simulink library "TripedLibraryMAX"  (R2021a)
settings: Solver=VariableStepAuto  StartTime=0.0  StopTime=10.0  RelTol=1e-3
undefined symbols (not defined in the file or in its folder): X, Y, Z
libraries: sm_lib x100, nesl_utility x31, $bdroot x27, robotlib x27, simulink x6
(part 6 of 6)

== matlab function TripedLibraryMAX/Simplified Model/Simplified TriPed (Kinematics)/Forward Kinematics/MATLAB Function ==
  data: Jointstate input; leg_pos output; LegNumber input
function leg_pos = fcn(Jointstate, LegNumber)
waym = Weg0(eul2quat(Jointstate(1:3).','XYZ'));
wayLLm = AddLegLinear(waym,Jointstate(4));
legstate = getPosfromHomogenMatrix(wayLLm);
leg_pos = transformCoordinateSystem(legstate(1),legstate(2),legstate(3),LegNumber);
end




function [new_pos] = transformCoordinateSystem(oldx,oldy,oldz,legNr)
% this function translates the x,y,z coordinates from the CCS into
% coordinates from a cooridnate system, which is like the ccs but rotated
% ,such that its x axis points towards the leg. This makes the inverse
% kinematics for the leg 1 & 2 easier.

angle = -legNr * 120 * pi/180;

newCoord = [cos(angle), -sin(angle), 0, 0; sin(angle), cos(angle), 0, 0; 0, 0, 1, 0; 0, 0, 0, 1]* [oldx;oldy;oldz;1];
newx = newCoord(1);
newy = newCoord(2);
newz = newCoord(3);

new_pos=[newx;newy;newz];
end

function [Weg0Mat] = Weg0(quat)
a0=quat(1);
b0=quat(2);
c0=quat(3);
d0=quat(4);
A1 = homogenTranslationMat(0.265,0,0.014);
A2 = makeRotHomogen(quatRotM(a0,b0,c0,d0));
Weg0Mat = A1 * A2;
end



function [ALeg] = AddLegLinear(A,theta0)
% this functions adds transformations from the Point P; which is the
% Endpoint from Weg0,1,2; to the FKS at the LegLinear part.
%{
Inputs:
A: Transformationmatrix, which describes Transformation from HKS to P, Use
Weg0.m for that.
theta0: angle [radians] for joint at MKS00
%}

A1 = homogenTranslationMat(1.640, 0, -0.037); % transform P to P0 (center of LegLinear rotation)

theta0 = -1* theta0 * 0.07/(2*pi*1.5); % gear constraint: transfrom theta at MKS00 to theta around P0, gear ratio is 0.07/(2*pi*1.5) [circumferences of the gears MCS00 / LL]
startingvalue = -0 * pi/180;
theta0 = theta0 + startingvalue; %offset, depending on which position the Leg is for theta at MKS00 is 0
A2 = makeRotHomogen(quatRotM(cos(theta0/2),0,sin(theta0/2),0)); % rotate theta [radians] around y-axis of P0

A3 = homogenTranslationMat(-1.500,0,0); %transform from P0-joint to FKS

ALeg = A*A1*A2*A3;
end

function [res] = homogenTranslationMat(x,y,z)
    %this function forms a Translation about a vector x,y,z in a homogenous
    %transformation matrix res. 
    res = [1 0 0 x; 0 1 0 y; 0 0 1 z; 0 0 0 1];
end

function [res] = makeRotHomogen(R)
% this function fills a Rotationmatrix to make it a homogen transformation
% matrix
res = [R(1,1) R(1,2) R(1,3) 0; R(2,1) R(2,2) R(2,3) 0; R(3,1) R(3,2) R(3,3) 0; 0 0 0 1];
end

function [res] = quatRotM(a,b,c,d) %%Todo: use a,b,c,d as inputs
    % this function calculates the quaternion rotation matrix for given
    % vector (kx,ky,kz) and angle theta
    res = [1-2*(c^2+d^2) 2*(b*c-d*a) 2*(b*d + c*a); 2*(b*c + d*a) 1-2*(b^2+d^2) 2*(c*d - b*a); 2*(b*d-c*a) 2*(c*d+b*a) 1-2*(b^2+c^2) ];
end




function [pos] = getPosfromHomogenMatrix(A)
    % this function returns the Vector by which a homogenous
    % Transformationmatrix shifts a Point
    x = A(1,4);
    y = A(2,4);
    z = A(3,4);

    pos=[x,y,z];
end

== matlab function TripedLibraryMAX/Examples/Spider Walking/Spider Gait ==
  settings: updateMethod=DISCRETE  sampleTime=0.01
  data: foot_0 output; swing_rad input; cmd_vel input; current_foot_pos input; current_state input; foot_1 output; foot_2 output; current_foot output; next_state output
function [foot_0,foot_1,foot_2,current_foot, next_state] = fcn( swing_rad,cmd_vel,current_foot_pos, current_state)


    initial_pos_0 = [0.4    ; 0       ;  -0]*1.2 + [0   ; 0       ; -0.6];
    initial_pos_1 = [-0.2025; -0.35074;  -0]*1.2 + [0   ; 0       ; -0.6];
    initial_pos_2 = [-0.2025;  0.35074;  -0]*1.2 + [0   ; 0       ; -0.6];
    
    init_angle_0  = mod(2*pi/360* 0   + 0*pi/2,2*pi);
    init_angle_1  = mod(2*pi/360* 240 + 0*pi/2,2*pi); %angle convention other direction for some reason
    init_angle_2  = mod(2*pi/360* 120 + 0*pi/2,2*pi);
    
    
    rel_step_angle= 60;
    final_angle_0 = mod(init_angle_0+2*pi/360*rel_step_angle,2*pi);
    final_angle_1 = mod(init_angle_1+2*pi/360*rel_step_angle,2*pi);
    final_angle_2 = mod(init_angle_2+2*pi/360*rel_step_angle,2*pi);
    
    current_foot= [0;0;0;0;0;0;0;0;0;0;0;0];
    next_state=current_state;

    
    persistent angle
    if isempty(angle)
        angle = [-2*pi;-2*pi;-2*pi];
    end

    
    persistent last_step_angle
    if isempty(last_step_angle)
        last_step_angle = [0;0;0];
    end
 
    persistent last_step_vel
    if isempty(last_step_vel)
        last_step_vel = [0;0;0;0;0;0];
    end

    persistent step_pos
    if isempty(step_pos)
        step_pos = [initial_pos_0;initial_pos_1;initial_pos_2];
    end
    
    
   [foot_0,current_foot(1:4) ,next_state(1),last_step_angle(1),last_step_vel(1:2),step_pos(1:3),angle(1)]  = single_leg_state_machine(initial_pos_0,last_step_vel(1:2),last_step_angle(1),current_state(1),current_foot_pos(1:4) ,step_pos(1:3),angle(1),swing_rad,cmd_vel,final_angle_0,init_angle_0);
   [foot_1,current_foot(5:8) ,next_state(2),last_step_angle(2),last_step_vel(3:4),step_pos(4:6),angle(2)]  = single_leg_state_machine(initial_pos_1,last_step_vel(3:4),last_step_angle(2),current_state(2),current_foot_pos(5:8) ,step_pos(4:6),angle(2),swing_rad,cmd_vel,final_angle_1,init_angle_1);  
   [foot_2,current_foot(9:12),next_state(3),last_step_angle(3),last_step_vel(5:6),step_pos(7:9),angle(3)]  = single_leg_state_machine(initial_pos_2,last_step_vel(5:6),last_step_angle(3),current_state(3),current_foot_pos(9:12),step_pos(7:9),angle(3),swing_rad,cmd_vel,final_angle_2,init_angle_2);


end

function [foot,current_liftoff,next_state,current_step,last_vel,step_pos,angle]=single_leg_state_machine(initial_pos,last_vel,prev_step,current_state,foot_pos,step_pos,last_angle,swing_rad,cmd_vel,stop_angle,start_angle)

timestep    = 0.01;
increment   = pi;
angle       = last_angle+increment*timestep;

height= 0.08;




[rad_walking_dir,velocity]=cart2pol(cmd_vel(1),cmd_vel(2));
walking_dir=rad_walking_dir/(2*pi)*360;
velocity = velocity/(2*pi);%/increment;

rel_step_angle = 60;%step_time/increment/timestep;
    



move_memory=angle-prev_step;

switch (current_state)
    case 0
        init_angle    = foot_pos(4);
        final_angle   = init_angle+2*pi/360*rel_step_angle;
        s = angdiff(angle,init_angle)/angdiff(final_angle,init_angle);
       
        
        if (s<=1)
            next_state = 0;
            current_step = prev_step+2.5*0.01;
        else
            next_state =1;
            current_step = angle;

        end
        
        est_trvl=  2*abs(angdiff(final_angle,init_angle)); %max((2*pi-move_memory)/increment,0);
        final_pos    = initial_pos+swing_rad*[cos(final_angle);sin(final_angle);0] + rotz(last_vel(2))*[1;0;0]*est_trvl*last_vel(1)/2;
        foot         = targeted_step(s,height,foot_pos(1:3),final_pos);
        current_liftoff = foot_pos; 
        step_pos  = initial_pos + rotz(last_vel(2))*[1;0;0]*est_trvl*last_vel(1)/2;

        
    case 1

        foot = step_pos+swing_rad*[cos(angle);sin(angle);0]-rotz(walking_dir)*[1;0;0]*move_memory*velocity;
        if (mod(angle,2*pi) >=start_angle) &&(mod(angle,2*pi) <=stop_angle) &&  norm(step_pos+swing_rad*[cos(angle);sin(angle);0]-foot) >=0.05
            next_state =0;
        else
            next_state = 1;
        end

        current_liftoff = [foot;angle];
        current_step = prev_step;
        last_vel = [velocity;walking_dir];
        
    % initialisation case to allow the simulation to settle
    case 2
        if angle >= 0
            next_state=1;
        else
            next_state=2;    
        end
        foot = initial_pos+(2*pi+angle)/(2*pi)*swing_rad*[cos(0);sin(0);0];
        current_liftoff = [foot;0]; 
        current_step = prev_step;
        
    otherwise
         next_state      = current_state;
         
         foot            = foot_pos(1:3);
         current_liftoff = foot_pos; 
         current_step = prev_step;
end
end


function foot_position=targeted_step(s,height,start,stop)
    relative_pos  = stop-start;
    [rot,len]   = cart2pol(relative_pos(1),relative_pos(2));
    foot_position = rotz(rot/(2*pi)*360)*step(s,len,height)+start;
end

function foot_position=step(s,length,height)

z  = height*exp(-(s-0.5)^2/0.01);%0.01

x = length/(1+exp(-20*(s-0.5)));
y = 0; 

foot_position = [x; y; z];
end

== matlab function TripedLibraryMAX/Full Model/ROS/Publishers/Publisher_leg_1/leg_1_extend_joint_pub/mergeValuesIntoRosMsg_test ==
  data: blankMessage input; position input; msg output Bus: SL_Bus_rosTest_sensor_msgs_JointState
function msg = assignNestedArrayMessages(blankMessage,position)
%#codegen

% To set datatype of MSG output:
%  1. Create buses for this model: robotics.ros.createSimulinkBus(gcs)
%  1. Click "Edit Data" in  Toolstrip to open "Ports and Data Manager"
%  2. Select MSG and set datatype to "Bus: SL_Bus_<modelname>_<messageType>"
% See: 
% http://www.mathworks.com/help/simulink/ug/create-structures-in-matlab-function-blocks.html

% blankMessage (geometry_msgs/PoseArray) consists of
%          Header: [1x1 Header]
%           Poses: variable-length array of geometry_msgs/Pose
%   Poses_SL_Info: struct with fields CurrentLength, ReceivedLength
%
% Poses is an array of "geometry_msgs/Pose", which consists of
%        Position: [1x1 Point]
%     Orientation: [1x1 Quaternion]
%
% Position is "geometry_msgs/Point", which consists of
%               X: 0
%               Y: 0
%               Z: 0
%
% Orientation is "geometry_msgs/Quaternion", which consists of
%               X: 0
%               Y: 0
%               Z: 0
%               W: 0

% Set the Poses array to have size 2, and modify the first two Pose
% elements

%blankMessage.Poses_SL_Info.CurrentLength = uint32(2);
%blankMessage.Poses(1).Position.X = x;
%blankMessage.Poses(1).Position.Y = y;
%blankMessage.Poses(2).Orientation.Z = z;

blankMessage.Position_SL_Info.CurrentLength = uint32(1);
%blankMessage.Velocity_SL_Info.CurrentLength = uint32(2);
%blankMessage.Effort_SL_Info.CurrentLength = uint32(2);
blankMessage.Position(1) = position;
%blankMessage.Velocity(2) = velocity;
%blankMessage.Position(2) = effort;

msg = blankMessage;

== matlab function TripedLibraryMAX/Full Model/ROS/Publishers/Publisher_leg_0/leg_0_swing_left_joint_pub/mergeValuesIntoRosMsg_test ==
  data: blankMessage input; position input; msg output Bus: SL_Bus_rosTest_sensor_msgs_JointState
function msg = assignNestedArrayMessages(blankMessage,position)
%#codegen

% To set datatype of MSG output:
%  1. Create buses for this model: robotics.ros.createSimulinkBus(gcs)
%  1. Click "Edit Data" in  Toolstrip to open "Ports and Data Manager"
%  2. Select MSG and set datatype to "Bus: SL_Bus_<modelname>_<messageType>"
% See: 
% http://www.mathworks.com/help/simulink/ug/create-structures-in-matlab-function-blocks.html

% blankMessage (geometry_msgs/PoseArray) consists of
%          Header: [1x1 Header]
%           Poses: variable-length array of geometry_msgs/Pose
%   Poses_SL_Info: struct with fields CurrentLength, ReceivedLength
%
% Poses is an array of "geometry_msgs/Pose", which consists of
%        Position: [1x1 Point]
%     Orientation: [1x1 Quaternion]
%
% Position is "geometry_msgs/Point", which consists of
%               X: 0
%               Y: 0
%               Z: 0
%
% Orientation is "geometry_msgs/Quaternion", which consists of
%               X: 0
%               Y: 0
%               Z: 0
%               W: 0

% Set the Poses array to have size 2, and modify the first two Pose
% elements

%blankMessage.Poses_SL_Info.CurrentLength = uint32(2);
%blankMessage.Poses(1).Position.X = x;
%blankMessage.Poses(1).Position.Y = y;
%blankMessage.Poses(2).Orientation.Z = z;

blankMessage.Position_SL_Info.CurrentLength = uint32(1);
%blankMessage.Velocity_SL_Info.CurrentLength = uint32(2);
%blankMessage.Effort_SL_Info.CurrentLength = uint32(2);
blankMessage.Position(1) = position;
%blankMessage.Velocity(2) = velocity;
%blankMessage.Position(2) = effort;

msg = blankMessage;

== matlab function TripedLibraryMAX/Full Model/ROS/Publishers/Publisher_leg_1/leg_1_swing_left_joint_pub/mergeValuesIntoRosMsg_test ==
  data: blankMessage input; position input; msg output Bus: SL_Bus_rosTest_sensor_msgs_JointState
function msg = assignNestedArrayMessages(blankMessage,position)
%#codegen

% To set datatype of MSG output:
%  1. Create buses for this model: robotics.ros.createSimulinkBus(gcs)
%  1. Click "Edit Data" in  Toolstrip to open "Ports and Data Manager"
%  2. Select MSG and set datatype to "Bus: SL_Bus_<modelname>_<messageType>"
% See: 
% http://www.mathworks.com/help/simulink/ug/create-structures-in-matlab-function-blocks.html

% blankMessage (geometry_msgs/PoseArray) consists of
%          Header: [1x1 Header]
%           Poses: variable-length array of geometry_msgs/Pose
%   Poses_SL_Info: struct with fields CurrentLength, ReceivedLength
%
% Poses is an array of "geometry_msgs/Pose", which consists of
%        Position: [1x1 Point]
%     Orientation: [1x1 Quaternion]
%
% Position is "geometry_msgs/Point", which consists of
%               X: 0
%               Y: 0
%               Z: 0
%
% Orientation is "geometry_msgs/Quaternion", which consists of
%               X: 0
%               Y: 0
%               Z: 0
%               W: 0

% Set the Poses array to have size 2, and modify the first two Pose
% elements

%blankMessage.Poses_SL_Info.CurrentLength = uint32(2);
%blankMessage.Poses(1).Position.X = x;
%blankMessage.Poses(1).Position.Y = y;
%blankMessage.Poses(2).Orientation.Z = z;

blankMessage.Position_SL_Info.CurrentLength = uint32(1);
%blankMessage.Velocity_SL_Info.CurrentLength = uint32(2);
%blankMessage.Effort_SL_Info.CurrentLength = uint32(2);
blankMessage.Position(1) = position;
%blankMessage.Velocity(2) = velocity;
%blankMessage.Position(2) = effort;

msg = blankMessage;

== matlab function TripedLibraryMAX/Simplified Model/Simplified TriPed (Kinematics)/Forward Kinematics/MATLAB Function1 ==
  data: Jointstate input; leg_pos output; LegNumber input
function leg_pos = fcn(Jointstate, LegNumber)
waym = Weg0(eul2quat(Jointstate(1:3).','XYZ'));
wayLLm = AddLegLinear(waym,Jointstate(4));
legstate = getPosfromHomogenMatrix(wayLLm);
leg_pos = transformCoordinateSystem(legstate(1),legstate(2),legstate(3),LegNumber);
end




function [new_pos] = transformCoordinateSystem(oldx,oldy,oldz,legNr)
% this function translates the x,y,z coordinates from the CCS into
% coordinates from a cooridnate system, which is like the ccs but rotated
% ,such that its x axis points towards the leg. This makes the inverse
% kinematics for the leg 1 & 2 easier.

angle = -legNr * 120 * pi/180;

newCoord = [cos(angle), -sin(angle), 0, 0; sin(angle), cos(angle), 0, 0; 0, 0, 1, 0; 0, 0, 0, 1]* [oldx;oldy;oldz;1];
newx = newCoord(1);
newy = newCoord(2);
newz = newCoord(3);

new_pos=[newx;newy;newz];
end

function [Weg0Mat] = Weg0(quat)
a0=quat(1);
b0=quat(2);
c0=quat(3);
d0=quat(4);
A1 = homogenTranslationMat(0.265,0,0.014);
A2 = makeRotHomogen(quatRotM(a0,b0,c0,d0));
Weg0Mat = A1 * A2;
end



function [ALeg] = AddLegLinear(A,theta0)
% this functions adds transformations from the Point P; which is the
% Endpoint from Weg0,1,2; to the FKS at the LegLinear part.
%{
Inputs:
A: Transformationmatrix, which describes Transformation from HKS to P, Use
Weg0.m for that.
theta0: angle [radians] for joint at MKS00
%}

A1 = homogenTranslationMat(1.640, 0, -0.037); % transform P to P0 (center of LegLinear rotation)

theta0 = -1* theta0 * 0.07/(2*pi*1.5); % gear constraint: transfrom theta at MKS00 to theta around P0, gear ratio is 0.07/(2*pi*1.5) [circumferences of the gears MCS00 / LL]
startingvalue = -0 * pi/180;
theta0 = theta0 + startingvalue; %offset, depending on which position the Leg is for theta at MKS00 is 0
A2 = makeRotHomogen(quatRotM(cos(theta0/2),0,sin(theta0/2),0)); % rotate theta [radians] around y-axis of P0

A3 = homogenTranslationMat(-1.500,0,0); %transform from P0-joint to FKS

ALeg = A*A1*A2*A3;
end

function [res] = homogenTranslationMat(x,y,z)
    %this function forms a Translation about a vector x,y,z in a homogenous
    %transformation matrix res. 
    res = [1 0 0 x; 0 1 0 y; 0 0 1 z; 0 0 0 1];
end

function [res] = makeRotHomogen(R)
% this function fills a Rotationmatrix to make it a homogen transformation
% matrix
res = [R(1,1) R(1,2) R(1,3) 0; R(2,1) R(2,2) R(2,3) 0; R(3,1) R(3,2) R(3,3) 0; 0 0 0 1];
end

function [res] = quatRotM(a,b,c,d) %%Todo: use a,b,c,d as inputs
    % this function calculates the quaternion rotation matrix for given
    % vector (kx,ky,kz) and angle theta
    res = [1-2*(c^2+d^2) 2*(b*c-d*a) 2*(b*d + c*a); 2*(b*c + d*a) 1-2*(b^2+d^2) 2*(c*d - b*a); 2*(b*d-c*a) 2*(c*d+b*a) 1-2*(b^2+c^2) ];
end




function [pos] = getPosfromHomogenMatrix(A)
    % this function returns the Vector by which a homogenous
    % Transformationmatrix shifts a Point
    x = A(1,4);
    y = A(2,4);
    z = A(3,4);

    pos=[x,y,z];
end

== matlab function TripedLibraryMAX/Simplified Model/Simplified TriPed (Kinematics)/Forward Kinematics/MATLAB Function2 ==
  data: Jointstate input; leg_pos output; LegNumber input
function leg_pos = fcn(Jointstate, LegNumber)
waym = Weg0(eul2quat(Jointstate(1:3).','XYZ'));
wayLLm = AddLegLinear(waym,Jointstate(4));
legstate = getPosfromHomogenMatrix(wayLLm);
leg_pos = transformCoordinateSystem(legstate(1),legstate(2),legstate(3),LegNumber);
end




function [new_pos] = transformCoordinateSystem(oldx,oldy,oldz,legNr)
% this function translates the x,y,z coordinates from the CCS into
% coordinates from a cooridnate system, which is like the ccs but rotated
% ,such that its x axis points towards the leg. This makes the inverse
% kinematics for the leg 1 & 2 easier.

angle = -legNr * 120 * pi/180;

newCoord = [cos(angle), -sin(angle), 0, 0; sin(angle), cos(angle), 0, 0; 0, 0, 1, 0; 0, 0, 0, 1]* [oldx;oldy;oldz;1];
newx = newCoord(1);
newy = newCoord(2);
newz = newCoord(3);

new_pos=[newx;newy;newz];
end

function [Weg0Mat] = Weg0(quat)
a0=quat(1);
b0=quat(2);
c0=quat(3);
d0=quat(4);
A1 = homogenTranslationMat(0.265,0,0.014);
A2 = makeRotHomogen(quatRotM(a0,b0,c0,d0));
Weg0Mat = A1 * A2;
end



function [ALeg] = AddLegLinear(A,theta0)
% this functions adds transformations from the Point P; which is the
% Endpoint from Weg0,1,2; to the FKS at the LegLinear part.
%{
Inputs:
A: Transformationmatrix, which describes Transformation from HKS to P, Use
Weg0.m for that.
theta0: angle [radians] for joint at MKS00
%}

A1 = homogenTranslationMat(1.640, 0, -0.037); % transform P to P0 (center of LegLinear rotation)

theta0 = -1* theta0 * 0.07/(2*pi*1.5); % gear constraint: transfrom theta at MKS00 to theta around P0, gear ratio is 0.07/(2*pi*1.5) [circumferences of the gears MCS00 / LL]
startingvalue = -0 * pi/180;
theta0 = theta0 + startingvalue; %offset, depending on which position the Leg is for theta at MKS00 is 0
A2 = makeRotHomogen(quatRotM(cos(theta0/2),0,sin(theta0/2),0)); % rotate theta [radians] around y-axis of P0

A3 = homogenTranslationMat(-1.500,0,0); %transform from P0-joint to FKS

ALeg = A*A1*A2*A3;
end

function [res] = homogenTranslationMat(x,y,z)
    %this function forms a Translation about a vector x,y,z in a homogenous
    %transformation matrix res. 
    res = [1 0 0 x; 0 1 0 y; 0 0 1 z; 0 0 0 1];
end

function [res] = makeRotHomogen(R)
% this function fills a Rotationmatrix to make it a homogen transformation
% matrix
res = [R(1,1) R(1,2) R(1,3) 0; R(2,1) R(2,2) R(2,3) 0; R(3,1) R(3,2) R(3,3) 0; 0 0 0 1];
end

function [res] = quatRotM(a,b,c,d) %%Todo: use a,b,c,d as inputs
    % this function calculates the quaternion rotation matrix for given
    % vector (kx,ky,kz) and angle theta
    res = [1-2*(c^2+d^2) 2*(b*c-d*a) 2*(b*d + c*a); 2*(b*c + d*a) 1-2*(b^2+d^2) 2*(c*d - b*a); 2*(b*d-c*a) 2*(c*d+b*a) 1-2*(b^2+c^2) ];
end




function [pos] = getPosfromHomogenMatrix(A)
    % this function returns the Vector by which a homogenous
    % Transformationmatrix shifts a Point
    x = A(1,4);
    y = A(2,4);
    z = A(3,4);

    pos=[x,y,z];
end

== matlab function TripedLibraryMAX/Full Model/ROS/Publishers/Publisher_leg_0/leg_0_swing_right_joint_pub/mergeValuesIntoRosMsg_test ==
  data: blankMessage input; position input; msg output Bus: SL_Bus_rosTest_sensor_msgs_JointState
function msg = assignNestedArrayMessages(blankMessage,position)
%#codegen

% To set datatype of MSG output:
%  1. Create buses for this model: robotics.ros.createSimulinkBus(gcs)
%  1. Click "Edit Data" in  Toolstrip to open "Ports and Data Manager"
%  2. Select MSG and set datatype to "Bus: SL_Bus_<modelname>_<messageType>"
% See: 
% http://www.mathworks.com/help/simulink/ug/create-structures-in-matlab-function-blocks.html

% blankMessage (geometry_msgs/PoseArray) consists of
%          Header: [1x1 Header]
%           Poses: variable-length array of geometry_msgs/Pose
%   Poses_SL_Info: struct with fields CurrentLength, ReceivedLength
%
% Poses is an array of "geometry_msgs/Pose", which consists of
%        Position: [1x1 Point]
%     Orientation: [1x1 Quaternion]
%
% Position is "geometry_msgs/Point", which consists of
%               X: 0
%               Y: 0
%               Z: 0
%
% Orientation is "geometry_msgs/Quaternion", which consists of
%               X: 0
%               Y: 0
%               Z: 0
%               W: 0

% Set the Poses array to have size 2, and modify the first two Pose
% elements

%blankMessage.Poses_SL_Info.CurrentLength = uint32(2);
%blankMessage.Poses(1).Position.X = x;
%blankMessage.Poses(1).Position.Y = y;
%blankMessage.Poses(2).Orientation.Z = z;

blankMessage.Position_SL_Info.CurrentLength = uint32(1);
%blankMessage.Velocity_SL_Info.CurrentLength = uint32(2);
%blankMessage.Effort_SL_Info.CurrentLength = uint32(2);
blankMessage.Position(1) = position;
%blankMessage.Velocity(2) = velocity;
%blankMessage.Position(2) = effort;

msg = blankMessage;
